simulink model "Simulatore_COMAU_UBHAND_IV_manipolazione_interna"  (Simulink 7.6)
settings: Solver=ode45  StartTime=0.0  StopTime=inf  RelTol=1e-3
values from neighbouring files (symbols this model uses):
  a_f = zeros(4,5)    [KinematicData.m]
  alpha_f = zeros(4,5)    [KinematicData.m]
  d_f = zeros(4,5)    [KinematicData.m]
  dc4_1 = 660    [KinematicData.m]
  T6HAND = [1 0 0 0;0 1 0 0; 0 0 1 357.48+95; 0 0 0 1]    [KinematicData.m]
  teta_d = atan(647.07/130)    [KinematicData.m]
  teta_f = zeros(4,5)    [KinematicData.m]
undefined symbols (not defined in the file or in its folder): a_c, a_w, alpha_c, alpha_w, d_c, d_w, Dt_fingers, teta_c
libraries: simulink x281, dspmtrx3 x171, aerolibutil x163, aerolibconvert2 x64, simulink_extras x46, vrlib x26, aerolibtransform2 x7, dspnetwork x1
(part 2 of 15)

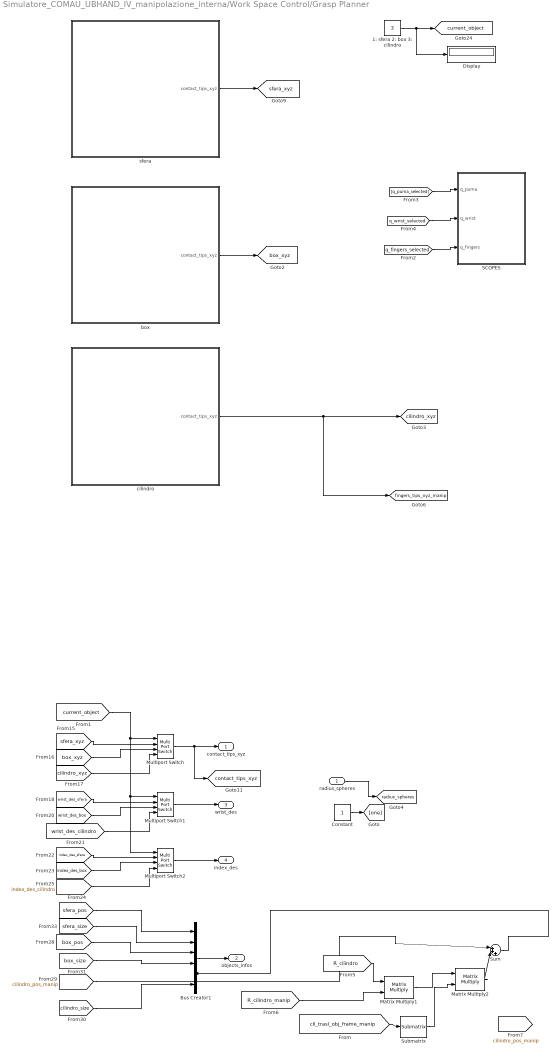
== system Simulatore_COMAU_UBHAND_IV_manipolazione_interna/Work Space Control/Grasp Planner ==
  radius_spheres : Inport
  1: sfera 2: box 3: cilindro : Constant  Value=3
  Bus Creator1 : BusCreator  Inputs=6
  Constant : Constant
  Display : Display
  Matrix Multiply1 : Product  Multiplication=Matrix(*)
  Matrix Multiply2 : Product  Multiplication=Matrix(*)
  Multiport Switch : MultiPortSwitch  zeroidx=off
  Multiport Switch1 : MultiPortSwitch  zeroidx=off
  Multiport Switch2 : MultiPortSwitch  zeroidx=off
  SCOPES : Subsystem
  Submatrix : dspmtrx3/Submatrix  RowSpan=Range of rows  RowStartMode=Index  RowStartIndex=1  RowEndMode=Index  RowEndIndex=3  ColSpan=One column  ColStartMode=Index  ColStartIndex=1  ColEndMode=Index  ColEndIndex=3
  Sum : Sum  Inputs=|+++
  box : Subsystem
  cilindro : Subsystem
  sfera : Subsystem
  contact_tips_xyz : Outport
  objects_infos : Outport  Port=2
  wrist_des : Outport  Port=3
  index_des : Outport  Port=4
  tag scope: box_xyz=global, cilindro_xyz=global, contact_tips_xyz=global, current_object=global, fingers_tips_xyz_manip=global, radius_spheres=global, sfera_xyz=global
  wires:
  Constant/1 -> [one]
  Multiport Switch/1 -> [contact_tips_xyz], contact_tips_xyz/1
  [sfera_xyz] -> Multiport Switch/2
  [box_xyz] -> Multiport Switch/3
  [cilindro_xyz] -> Multiport Switch/4
  radius_spheres/1 -> [radius_spheres]
  [sfera_pos] -> Bus Creator1/1
  [sfera_size] -> Bus Creator1/2
  [box_pos] -> Bus Creator1/3
  [box_size] -> Bus Creator1/4
  [cilindro_size] -> Bus Creator1/6
  Bus Creator1/1 -> objects_infos/1
  Multiport Switch1/1 -> wrist_des/1
  [wrist_des_sfera] -> Multiport Switch1/2
  [wrist_des_box] -> Multiport Switch1/3
  [wrist_des_cilindro] -> Multiport Switch1/4
  Multiport Switch2/1 -> index_des/1
  [index_des_sfera] -> Multiport Switch2/2
  [index_des_box] -> Multiport Switch2/3
  [index_des_cilindro] -> Multiport Switch2/4
  sfera/1(contact_tips_xyz) -> [sfera_xyz]
  box/1(contact_tips_xyz) -> [box_xyz]
  1: sfera 2: box 3: cilindro/1 -> [current_object], Display/1
  [current_object] -> Multiport Switch/1, Multiport Switch1/1, Multiport Switch2/1
  [q_puma_selected] -> SCOPES/1(q_puma)
  [q_wrist_selected] -> SCOPES/2(q_wrist)
  [q_fingers_selected] -> SCOPES/3(q_fingers)
  cilindro/1(contact_tips_xyz) -> [cilindro_xyz], [fingers_tips_xyz_manip]
  [cil_trasl_obj_frame_manip] -> Submatrix/1
  Matrix Multiply2/1 -> Sum/2
  [cilindro_pos_manip] -> Sum/1
  Sum/1 -> Bus Creator1/5
  Submatrix/1 -> Matrix Multiply2/2
  [R_cilindro_manip] -> Matrix Multiply1/2
  [R_cilindro] -> Matrix Multiply1/1
  Matrix Multiply1/1 -> Matrix Multiply2/1

== system Simulatore_COMAU_UBHAND_IV_manipolazione_interna/Work Space Control/Inverse_kinematic ==
  note: punti di contatto nel sistema di riferimento dito
  "fingers_tips_xyz " : Inport
  wrist_angles_des : Inport  Port=2
  index_angles_des : Inport  Port=3
  Demux : Demux  Outputs=5
  Demux1 : Demux  Outputs=20
  Display : Display
  Inverse_kinematic_comau : Subsystem
  Inverse_kinematic_hand : Subsystem
  Multiport Switch3 : MultiPortSwitch  Inputs=2  zeroidx=off
  Mux{,1,3..5} (5 alike) : Mux
  Mux2 : Mux  Inputs=5
  Mux6 : Mux  Inputs=5
  Submatrix : dspmtrx3/Submatrix  RowSpan=Range of rows  RowStartMode=Index  RowStartIndex=1  RowEndMode=Index  RowEndIndex=3  ColSpan=One column  ColStartMode=Index  ColStartIndex=1  ColEndMode=Index  ColEndIndex=3
  Tf0 : Subsystem
  teta[1..4] : Display
  teta[1..4]1 : Display
  wrist_angles_des1 : InportShadow  Port=2
  q[1..6] : Outport
  finger_joints references : Outport  Port=2
  T_0H : Outport  Port=3
  wires:
  Inverse_kinematic_comau/1(q[1..6]) -> q[1..6]/1
  wrist_angles_des/1 -> Inverse_kinematic_comau/2(wrist_angles_des)
  index_angles_des/1 -> Inverse_kinematic_comau/3(index_angles_des)
  Mux2/1 -> finger_joints references/1
  Inverse_kinematic_comau/2(T_0H) -> Tf0/1(T_0HAND), T_0H/1
  Tf0/1(fingers_references xyz fingers_references 1) -> [fingers_xyz_fing_ref], teta[1..4]1/1
  [fingers_xyz_fing_ref] -> Multiport Switch3/3
  Multiport Switch3/1 -> Demux/1
  Demux/1 -> Inverse_kinematic_hand/1(xyz_thumb)
  Inverse_kinematic_hand/1(Thumb) -> Mux2/1
  [fingers_tips_xyz] -> Multiport Switch3/2
  [fingers_tips_xyz] -> Tf0/3(fingers_references_xyz 0)
  "fingers_tips_xyz "/1 -> Demux1/1
  Demux1/1 -> Mux/1
  Demux1/2 -> Mux/2
  Demux1/3 -> Mux/3
  Demux1/4 -> Mux/4
  Demux1/5 -> Mux1/1
  Demux1/6 -> Mux1/2
  Demux1/7 -> Mux1/3
  Demux1/9 -> Mux3/1
  Demux1/10 -> Mux3/2
  Demux1/11 -> Mux3/3
  Demux1/12 -> Mux3/4
  Demux1/13 -> Mux4/1
  Demux1/14 -> Mux4/2
  Demux1/15 -> Mux4/3
  Demux1/16 -> Mux4/4
  Demux1/17 -> Mux5/1
  Demux1/18 -> Mux5/2
  Demux1/19 -> Mux5/3
  Demux1/20 -> Mux5/4
  Mux1/1 -> Mux6/2
  Mux/1 -> Mux6/1
  Mux6/1 -> [fingers_tips_xyz]
  wrist_angles_des1/1 -> Tf0/2(wrist_angles[1..2])
  [reference_mode] -> Multiport Switch3/1
  Mux3/1 -> Mux6/3
  Mux4/1 -> Mux6/4
  Mux5/1 -> Mux6/5
  Demux/5 -> Inverse_kinematic_hand/5(xyz_little)
  Demux/4 -> Inverse_kinematic_hand/4(xyz_ring)
  Demux/3 -> Inverse_kinematic_hand/3(xyz_middle)
  Demux/2 -> Inverse_kinematic_hand/2(xyz_index)
  Demux1/8 -> Mux1/4
  Submatrix/1 -> Inverse_kinematic_comau/1(end_effector_xyz), Display/1
  Inverse_kinematic_hand/5(Little) -> Mux2/5
  Inverse_kinematic_hand/4(Ring) -> Mux2/4
  Inverse_kinematic_hand/3(Middle) -> Mux2/3
  Inverse_kinematic_hand/2(Index) -> Mux2/2, teta[1..4]/1
  [index_xyz_0] -> Submatrix/1

== system Simulatore_COMAU_UBHAND_IV_manipolazione_interna/Work Space Control/Piano di lavoro ==
  Constant : Constant
  Mux1 : Mux  Inputs=3
  Mux2 : Mux  Inputs=3
  x : simulink/Math Operations/Slider Gain  low=-1000  gain=1600  high=2000
  x_size : simulink/Math Operations/Slider Gain  low=-1000  gain=3000  high=3000
  y : simulink/Math Operations/Slider Gain  low=-1000  gain=-120  high=1000
  y_size : simulink/Math Operations/Slider Gain  low=-1000  gain=40  high=1000
  z : simulink/Math Operations/Slider Gain  low=-1000  gain=250  high=5010
  z_size : simulink/Math Operations/Slider Gain  low=-1000  gain=1800  high=5010
  tag scope: piano_size=global, piano_xyz=global
  wires:
  [one] -> x/1
  x/1 -> Mux2/1
  [one] -> y/1
  [one] -> z/1
  z/1 -> Mux2/3
  y/1 -> Mux2/2
  [one] -> x_size/1
  x_size/1 -> Mux1/1
  [one] -> y_size/1
  [one] -> z_size/1
  z_size/1 -> Mux1/3
  y_size/1 -> Mux1/2
  Mux2/1 -> [piano_xyz]
  Mux1/1 -> [piano_size]
  Constant/1 -> [one]

== system Simulatore_COMAU_UBHAND_IV_manipolazione_interna/Work Space Control/R_end_effector ==
  alpha,beta,gamma : Inport
  Demux : Demux  Outputs=3
  Matrix Multiply3 : Product  Multiplication=Matrix(*)
  Matrix Multiply4 : Product  Multiplication=Matrix(*)
  rot_x : Subsystem
  rot_y : Subsystem
  rot_z : Subsystem
  R_end_effector : Outport
  wires:
  Matrix Multiply4/1 -> R_end_effector/1
  Matrix Multiply3/1 -> Matrix Multiply4/1
  rot_z/1(rotz) -> Matrix Multiply4/2
  rot_y/1(roty) -> Matrix Multiply3/2
  rot_x/1(rotx) -> Matrix Multiply3/1
  Demux/1 -> rot_x/1(alpha)
  Demux/2 -> rot_y/1(beta)
  Demux/3 -> rot_z/1(gamma)
  alpha,beta,gamma/1 -> Demux/1

== system Simulatore_COMAU_UBHAND_IV_manipolazione_interna/Work Space Control/Step Signals fingers_wrist ==
  All_fingers_together_back_forward : Constant  Value=80
  All_fingers_together_medial : Constant  Value=80
  All_fingers_together_proximal_left_right : Constant  Value=0
  Index_medial1 : Constant  Value=30
  Index_proximal_back_forward1 : Constant  Value=45
  Index_proximal_left_right1 : Constant  Value=0
  Little_medial : Constant  Value=0
  Little_proximal_back_forward : Constant  Value=0
  Little_proximal_left_right : Constant  Value=0
  Middle_medial : Constant  Value=30
  Middle_proximal_back_forward : Constant  Value=45
  Middle_proximal_left_right : Constant  Value=0
  Mux{1..5,7} (6 alike) : Mux  Inputs=3
  Mux8 : Mux  Inputs=5
  Mux9 : Mux  Inputs=5
  Ring_medial : Constant  Value=0
  Ring_proximal_back_forward : Constant  Value=0
  Ring_proximal_left_right : Constant  Value=0
  Switch wrist : simulink/Signal Routing/Manual Switch  sw=0  action=0  varsize=off
  Thumb_medial : Constant  Value=50
  Thumb_proximal_back_forward : Constant  Value=50
  Thumb_proximal_left_right : Constant  Value=50
  fingers_angles : Outport
  wires:
  Middle_proximal_left_right/1 -> Mux3/1
  Middle_medial/1 -> Mux3/3
  Middle_proximal_back_forward/1 -> Mux3/2
  Ring_proximal_left_right/1 -> Mux4/1
  Ring_medial/1 -> Mux4/3
  Ring_proximal_back_forward/1 -> Mux4/2
  Little_proximal_left_right/1 -> Mux5/1
  Little_medial/1 -> Mux5/3
  Little_proximal_back_forward/1 -> Mux5/2
  Mux1/1 -> [thumb], Mux9/1
  Thumb_proximal_left_right/1 -> Mux1/1
  Thumb_medial/1 -> Mux1/3
  Thumb_proximal_back_forward/1 -> Mux1/2
  Mux3/1 -> [index]
  Mux4/1 -> [ring]
  Mux5/1 -> [little]
  Mux7/1 -> Mux9/3, Mux9/2, Mux9/4, Mux9/5
  All_fingers_together_proximal_left_right/1 -> Mux7/1
  All_fingers_together_medial/1 -> Mux7/3
  All_fingers_together_back_forward/1 -> Mux7/2
  Mux9/1 -> Switch wrist/2
  Mux8/1 -> Switch wrist/1
  Switch wrist/1 -> fingers_angles/1
  [thumb] -> Mux8/1
  Index_proximal_left_right1/1 -> Mux2/1
  Index_medial1/1 -> Mux2/3
  Index_proximal_back_forward1/1 -> Mux2/2
  Mux2/1 -> [middle]
  [little] -> Mux8/5
  [ring] -> Mux8/4
  [middle] -> Mux8/3
  [index] -> Mux8/2

== system Simulatore_COMAU_UBHAND_IV_manipolazione_interna/Work Space Control/posa_selection ==
  Index_medial_riposo : Constant  Value=0
  Index_proximal_back_forward_riposo : Constant  Value=0
  Index_proximal_left_right_riposo : Constant  Value=0
  Multiport Switch{,1..3} (4 alike) : MultiPortSwitch  Inputs=2  zeroidx=off
  Mux : Mux  Inputs=5
  Mux3 : Mux  Inputs=2
  Mux4 : Mux  Inputs=3
  Mux5 : Mux  Inputs=5
  fingers_rest_angles : Constant  Value=[ 0 0 0]
  wrist_back_forward_riposo : simulink/Math Operations/Slider Gain  low=-180  gain=0  high=180
  wrist_left_right_riposo : simulink/Math Operations/Slider Gain  low=-180  gain=0  high=180
  q_wrist : Outport
  q_fingers : Outport  Port=2
  tag scope: index_angles_des_C=global, wrist_angles_des_C=global
  wires:
  [one] -> wrist_left_right_riposo/1
  wrist_left_right_riposo/1 -> Mux3/1
  [one] -> wrist_back_forward_riposo/1
  wrist_back_forward_riposo/1 -> Mux3/2
  Index_proximal_left_right_riposo/1 -> Mux4/1
  Index_medial_riposo/1 -> Mux4/3
  Index_proximal_back_forward_riposo/1 -> Mux4/2
  [q_thumb] -> Mux/1
  [q_middle] -> Mux/3
  [q_ring] -> Mux/4
  [q_little] -> Mux/5
  fingers_rest_angles/1 -> Mux5/1, Mux5/3, Mux5/4, Mux5/5
  Mux4/1 -> Mux5/2
  Mux/1 -> Multiport Switch1/2
  Mux5/1 -> Multiport Switch1/3
  Multiport Switch1/1 -> q_fingers/1
  Mux3/1 -> Multiport Switch/3
  Multiport Switch/1 -> q_wrist/1
  [wrist_angles_des_C_2] -> Multiport Switch2/3
  [wrist_angles_des_C_1] -> Multiport Switch2/2
  Multiport Switch2/1 -> Multiport Switch/2, [wrist_angles_des_C]
  [index_angles_des_C_1] -> Multiport Switch3/2
  Multiport Switch3/1 -> [index_angles_des_C]
  [reference_mode] -> Multiport Switch2/1
  [reference_mode] -> Multiport Switch3/1
  [index_angles_des_C_2] -> Multiport Switch3/3
  [posa_selection] -> Multiport Switch/1, Multiport Switch1/1
  [q_index] -> Mux/2

== system Simulatore_COMAU_UBHAND_IV_manipolazione_interna/Work Space Control/wrist index angles des2 ==
  Constant : Constant
  Index_medial_des : simulink/Math Operations/Slider Gain  low=0  gain=30  high=110
  Index_proximal_back_forward_des : simulink/Math Operations/Slider Gain  low=0  gain=15  high=90
  Index_proximal_left_right_des : simulink/Math Operations/Slider Gain  low=-180  gain=20  high=180
  Mux1 : Mux  Inputs=2
  Mux2 : Mux  Inputs=3
  wrist_back_forward_des : simulink/Math Operations/Slider Gain  low=-180  gain=0  high=180
  wrist_left_right_des : simulink/Math Operations/Slider Gain  low=-180  gain=0  high=180
  wrist_des : Outport
  index_des : Outport  Port=2
  wires:
  Constant/1 -> [one]
  Mux1/1 -> wrist_des/1
  Mux2/1 -> index_des/1
  Index_proximal_back_forward_des/1 -> Mux2/2
  Index_medial_des/1 -> Mux2/3
  Index_proximal_left_right_des/1 -> Mux2/1
  wrist_back_forward_des/1 -> Mux1/2
  [one] -> wrist_back_forward_des/1
  wrist_left_right_des/1 -> Mux1/1
  [one] -> wrist_left_right_des/1
  [one] -> Index_proximal_left_right_des/1
  [one] -> Index_proximal_back_forward_des/1
  [one] -> Index_medial_des/1

== system Simulatore_COMAU_UBHAND_IV_manipolazione_interna/Joint Space Control/Direct_kinematic2/Direct_kinematic_Comau ==
  q[1..6] : Inport
  Demux3 : Demux  Outputs=6
  Matrix Multiply : Product  Multiplication=Matrix(*)
  Mux : Mux  Inputs=3
  Mux2 : Mux  Inputs=3
  T_0_3 : Subsystem
  T_3_6 : Subsystem
  T_0_6 : Outport
  wires:
  Demux3/1 -> Mux/1
  Demux3/2 -> Mux/2
  Demux3/3 -> Mux/3
  Mux/1 -> T_0_3/1(q[1..3])
  Demux3/4 -> Mux2/1
  Demux3/5 -> Mux2/2
  Demux3/6 -> Mux2/3
  Mux2/1 -> T_3_6/1(q[1..3])
  T_3_6/1(T_3_6) -> Matrix Multiply/2
  q[1..6]/1 -> Demux3/1
  T_0_3/1(T_0_3) -> Matrix Multiply/1
  Matrix Multiply/1 -> T_0_6/1

== system Simulatore_COMAU_UBHAND_IV_manipolazione_interna/Joint Space Control/Direct_kinematic2/Direct_kinematic_hand ==
  Joint_references : Inport
  T_08 : Inport  Port=2
  Constant : Constant  Value=Dt_fingers(1,1).T_w2_finger_base  VectorParams1D=off
  Constant1 : Constant  Value=Dt_fingers(1,2).T_w2_finger_base  VectorParams1D=off
  Constant2 : Constant  Value=Dt_fingers(1,3).T_w2_finger_base  VectorParams1D=off
  Constant3 : Constant  Value=Dt_fingers(1,4).T_w2_finger_base  VectorParams1D=off
  Constant4 : Constant  Value=Dt_fingers(1,5).T_w2_finger_base  VectorParams1D=off
  Demux : Demux  Outputs=5
  Direct_kinematic_index : Subsystem
  Direct_kinematic_little : Subsystem
  Direct_kinematic_middle : Subsystem
  Direct_kinematic_ring : Subsystem
  Direct_kinematic_thumb : Subsystem
  Matrix Multiply{,1..4} (5 alike) : Product  Multiplication=Matrix(*)
  xyz{,1..4} (5 alike) : dspmtrx3/Submatrix  RowSpan=Range of rows  RowStartMode=Index  RowStartIndex=1  RowEndMode=Index  RowEndIndex=4  ColSpan=One column  ColStartMode=Index  ColStartIndex=4  ColEndMode=Index  ColEndIndex=3
  T_finger_tip_thumb : Outport
  T_finger_tip_index : Outport  Port=2
  T_finger_tip_middle : Outport  Port=3
  T_finger_tip_ring : Outport  Port=4
  T_finger_tip_little : Outport  Port=5
  wires:
  T_08/1 -> Matrix Multiply/1, Matrix Multiply1/1, Matrix Multiply2/1, Matrix Multiply3/1, Matrix Multiply4/1
  Constant/1 -> Matrix Multiply/2
  Constant1/1 -> Matrix Multiply1/2
  Constant2/1 -> Matrix Multiply2/2
  Constant3/1 -> Matrix Multiply3/2
  Constant4/1 -> Matrix Multiply4/2
  Matrix Multiply/1 -> Direct_kinematic_thumb/2(T_0f)
  Matrix Multiply1/1 -> Direct_kinematic_index/2(T_0f)
  Matrix Multiply2/1 -> Direct_kinematic_middle/2(T_0f)
  Matrix Multiply3/1 -> Direct_kinematic_ring/2(T_0f)
  Matrix Multiply4/1 -> Direct_kinematic_little/2(T_0f)
  Joint_references/1 -> Demux/1
  Demux/1 -> Direct_kinematic_thumb/1(Joints_reference)
  Demux/2 -> Direct_kinematic_index/1(Joints_reference)
  Demux/3 -> Direct_kinematic_middle/1(Joints_reference)
  Demux/4 -> Direct_kinematic_ring/1(Joints_reference)
  Demux/5 -> Direct_kinematic_little/1(Joints_reference)
  Direct_kinematic_index/1(T_finger_tip) -> xyz1/1
  Direct_kinematic_middle/1(T_finger_tip) -> xyz2/1
  Direct_kinematic_ring/1(T_finger_tip) -> xyz3/1
  Direct_kinematic_little/1(T_finger_tip) -> xyz4/1
  Direct_kinematic_thumb/1(T_finger_tip) -> xyz/1
  xyz1/1 -> T_finger_tip_index/1
  xyz/1 -> T_finger_tip_thumb/1
  xyz2/1 -> T_finger_tip_middle/1
  xyz3/1 -> T_finger_tip_ring/1
  xyz4/1 -> T_finger_tip_little/1

== system Simulatore_COMAU_UBHAND_IV_manipolazione_interna/Joint Space Control/Direct_kinematic2/Direct_kinematic_wrist ==
  wrist_angles[1..2] : Inport
  T_0HAND : Inport  Port=2
  Demux3 : Demux  Outputs=2
  Mux : Mux  Inputs=2
  T_0_8 : Subsystem
  T_08 : Outport
  wires:
  T_0_8/1(T_08) -> T_08/1
  wrist_angles[1..2]/1 -> Demux3/1
  Mux/1 -> T_0_8/1(wrist_angles[1..2])
  Demux3/2 -> Mux/2
  Demux3/1 -> Mux/1
  T_0HAND/1 -> T_0_8/2(T_0HAND)

== system Simulatore_COMAU_UBHAND_IV_manipolazione_interna/SCOPES/Direct_kinematic2/Direct_kinematic_Comau ==
  q[1..6] : Inport
  Demux3 : Demux  Outputs=6
  Matrix Multiply : Product  Multiplication=Matrix(*)
  Mux : Mux  Inputs=3
  Mux2 : Mux  Inputs=3
  T_0_3 : Subsystem
  T_3_6 : Subsystem
  T_0_6 : Outport
  wires:
  Demux3/1 -> Mux/1
  Demux3/2 -> Mux/2
  Demux3/3 -> Mux/3
  Mux/1 -> T_0_3/1(q[1..3])
  Demux3/4 -> Mux2/1
  Demux3/5 -> Mux2/2
  Demux3/6 -> Mux2/3
  Mux2/1 -> T_3_6/1(q[1..3])
  T_3_6/1(T_3_6) -> Matrix Multiply/2
  q[1..6]/1 -> Demux3/1
  T_0_3/1(T_0_3) -> Matrix Multiply/1
  Matrix Multiply/1 -> T_0_6/1

== system Simulatore_COMAU_UBHAND_IV_manipolazione_interna/SCOPES/Direct_kinematic2/Direct_kinematic_hand ==
  Joint_references : Inport
  T_08 : Inport  Port=2
  Constant : Constant  Value=Dt_fingers(1,1).T_w2_finger_base  VectorParams1D=off
  Constant1 : Constant  Value=Dt_fingers(1,2).T_w2_finger_base  VectorParams1D=off
  Constant2 : Constant  Value=Dt_fingers(1,3).T_w2_finger_base  VectorParams1D=off
  Constant3 : Constant  Value=Dt_fingers(1,4).T_w2_finger_base  VectorParams1D=off
  Constant4 : Constant  Value=Dt_fingers(1,5).T_w2_finger_base  VectorParams1D=off
  Demux : Demux  Outputs=5
  Direct_kinematic_index : Subsystem
  Direct_kinematic_little : Subsystem
  Direct_kinematic_middle : Subsystem
  Direct_kinematic_ring : Subsystem
  Direct_kinematic_thumb : Subsystem
  Matrix Multiply{,1..4} (5 alike) : Product  Multiplication=Matrix(*)
  xyz{,1..4} (5 alike) : dspmtrx3/Submatrix  RowSpan=Range of rows  RowStartMode=Index  RowStartIndex=1  RowEndMode=Index  RowEndIndex=4  ColSpan=One column  ColStartMode=Index  ColStartIndex=4  ColEndMode=Index  ColEndIndex=3
  T_finger_tip_thumb : Outport
  T_finger_tip_index : Outport  Port=2
  T_finger_tip_middle : Outport  Port=3
  T_finger_tip_ring : Outport  Port=4
  T_finger_tip_little : Outport  Port=5
  T_thumb_base : Outport  Port=6
  tag scope: T_0_little=global, T_0_middle=global, T_0_ring=global, T_0_thumb=global
  wires:
  T_08/1 -> Matrix Multiply/1, Matrix Multiply1/1, Matrix Multiply2/1, Matrix Multiply3/1, Matrix Multiply4/1
  Constant/1 -> Matrix Multiply/2
  Constant1/1 -> Matrix Multiply1/2
  Constant2/1 -> Matrix Multiply2/2
  Constant3/1 -> Matrix Multiply3/2
  Constant4/1 -> Matrix Multiply4/2
  Matrix Multiply/1 -> Direct_kinematic_thumb/2(T_0f), [T_0_thumb]
  Matrix Multiply1/1 -> Direct_kinematic_index/2(T_0f)
  Matrix Multiply2/1 -> Direct_kinematic_middle/2(T_0f), [T_0_middle]
  Matrix Multiply3/1 -> Direct_kinematic_ring/2(T_0f), [T_0_ring]
  Matrix Multiply4/1 -> Direct_kinematic_little/2(T_0f), [T_0_little]
  Joint_references/1 -> Demux/1
  Demux/1 -> Direct_kinematic_thumb/1(Joints_reference)
  Demux/2 -> Direct_kinematic_index/1(Joints_reference)
  Demux/3 -> Direct_kinematic_middle/1(Joints_reference)
  Demux/4 -> Direct_kinematic_ring/1(Joints_reference)
  Demux/5 -> Direct_kinematic_little/1(Joints_reference)
  Direct_kinematic_index/1(T_finger_tip) -> xyz1/1
  Direct_kinematic_middle/1(T_finger_tip) -> xyz2/1
  Direct_kinematic_ring/1(T_finger_tip) -> xyz3/1
  Direct_kinematic_little/1(T_finger_tip) -> xyz4/1
  Direct_kinematic_thumb/1(T_finger_tip) -> xyz/1
  Direct_kinematic_thumb/2(T_thumb_base) -> T_thumb_base/1
  xyz1/1 -> T_finger_tip_index/1
  xyz/1 -> T_finger_tip_thumb/1
  xyz2/1 -> T_finger_tip_middle/1
  xyz3/1 -> T_finger_tip_ring/1
  xyz4/1 -> T_finger_tip_little/1

== system Simulatore_COMAU_UBHAND_IV_manipolazione_interna/SCOPES/Direct_kinematic2/Direct_kinematic_wrist ==
  wrist_angles[1..2] : Inport
  T_0HAND : Inport  Port=2
  Demux3 : Demux  Outputs=2
  Mux : Mux  Inputs=2
  T_0_8 : Subsystem
  T_08 : Outport
  wires:
  T_0_8/1(T_08) -> T_08/1
  wrist_angles[1..2]/1 -> Demux3/1
  Mux/1 -> T_0_8/1(wrist_angles[1..2])
  Demux3/2 -> Mux/2
  Demux3/1 -> Mux/1
  T_0HAND/1 -> T_0_8/2(T_0HAND)

== system Simulatore_COMAU_UBHAND_IV_manipolazione_interna/VISUALIZATION/Subsystem/sphere_xyz1 ==
  xyz_0 : Inport
  Constant : Constant  Value=0
  Constant1 : Constant  Value=0
  Constant2 : Constant  Value=0
  Demux : Demux  Outputs=3
  Display : Display
  Display1 : Display
  Gain : Gain  Gain=-1
  Gain1 : Gain  Gain=-1
  Gain2 : Gain
  Mux : Mux  Inputs=3
  Sum : Sum
  Sum1 : Sum
  Sum2 : Sum
  xyz_vrl : Outport
  wires:
  xyz_0/1 -> Display1/1, Demux/1
  Demux/1 -> Sum/2
  Constant/1 -> Sum/1
  Demux/3 -> Sum2/2
  Constant2/1 -> Sum2/1
  Demux/2 -> Sum1/2
  Constant1/1 -> Sum1/1
  Mux/1 -> xyz_vrl/1, Display/1
  Sum/1 -> Gain/1
  Sum1/1 -> Gain1/1
  Sum2/1 -> Gain2/1
  Gain/1 -> Mux/3
  Gain2/1 -> Mux/2
  Gain1/1 -> Mux/1

== system Simulatore_COMAU_UBHAND_IV_manipolazione_interna/VISUALIZATION/Subsystem1/box_xyz ==
  xyz_0 : Inport
  Constant : Constant  Value=0
  Constant1 : Constant  Value=0
  Constant2 : Constant  Value=0
  Demux : Demux  Outputs=3
  Display : Display
  Display1 : Display
  Gain : Gain  Gain=-1
  Gain1 : Gain  Gain=-1
  Gain2 : Gain
  Mux : Mux  Inputs=3
  Sum : Sum
  Sum1 : Sum
  Sum2 : Sum
  xyz_vrl : Outport
  wires:
  xyz_0/1 -> Display1/1, Demux/1
  Demux/1 -> Sum/2
  Constant/1 -> Sum/1
  Demux/3 -> Sum2/2
  Constant2/1 -> Sum2/1
  Demux/2 -> Sum1/2
  Constant1/1 -> Sum1/1
  Mux/1 -> xyz_vrl/1, Display/1
  Sum/1 -> Gain/1
  Sum1/1 -> Gain1/1
  Sum2/1 -> Gain2/1
  Gain/1 -> Mux/3
  Gain2/1 -> Mux/2
  Gain1/1 -> Mux/1

== system Simulatore_COMAU_UBHAND_IV_manipolazione_interna/VISUALIZATION/Subsystem1/cilindro_xyz ==
  xyz_0 : Inport
  Constant : Constant  Value=0
  Constant1 : Constant  Value=0
  Constant2 : Constant  Value=0
  Demux : Demux  Outputs=3
  Display : Display
  Display1 : Display
  Gain : Gain  Gain=-1
  Gain1 : Gain  Gain=-1
  Gain2 : Gain
  Mux : Mux  Inputs=3
  Sum : Sum
  Sum1 : Sum
  Sum2 : Sum
  xyz_vrl : Outport
  wires:
  Gain1/1 -> Mux/1
  Gain2/1 -> Mux/2
  Gain/1 -> Mux/3
  Sum2/1 -> Gain2/1
  Sum1/1 -> Gain1/1
  Sum/1 -> Gain/1
  Mux/1 -> Display/1, xyz_vrl/1
  Constant1/1 -> Sum1/1
  Demux/2 -> Sum1/2
  Constant2/1 -> Sum2/1
  Demux/3 -> Sum2/2
  Constant/1 -> Sum/1
  Demux/1 -> Sum/2
  xyz_0/1 -> Demux/1, Display1/1

== system Simulatore_COMAU_UBHAND_IV_manipolazione_interna/VISUALIZATION/Subsystem1/sphere_xyz ==
  xyz_0 : Inport
  Constant : Constant  Value=0
  Constant1 : Constant  Value=0
  Constant2 : Constant  Value=0
  Demux : Demux  Outputs=3
  Display : Display
  Display1 : Display
  Gain : Gain  Gain=-1
  Gain1 : Gain  Gain=-1
  Gain2 : Gain
  Mux : Mux  Inputs=3
  Sum : Sum
  Sum1 : Sum
  Sum2 : Sum
  xyz_vrl : Outport
  wires:
  Gain1/1 -> Mux/1
  Gain2/1 -> Mux/2
  Gain/1 -> Mux/3
  Sum2/1 -> Gain2/1
  Sum1/1 -> Gain1/1
  Sum/1 -> Gain/1
  Mux/1 -> Display/1, xyz_vrl/1
  Constant1/1 -> Sum1/1
  Demux/2 -> Sum1/2
  Constant2/1 -> Sum2/1
  Demux/3 -> Sum2/2
  Constant/1 -> Sum/1
  Demux/1 -> Sum/2
  xyz_0/1 -> Demux/1, Display1/1

== system Simulatore_COMAU_UBHAND_IV_manipolazione_interna/VISUALIZATION/Subsystem3/Subsystem1 ==
  TOHAND : Inport
  Demux8 : Demux
  Demux9 : Demux
  Gain : Gain  Gain=-1
  Gain1 : Gain  Gain=-1
  Mux : Mux
  Mux1 : Mux
  Rotation Matrix to VRML Rotation : vrlib/Utilities/Rotation Matrix to VRML Rotation  maxzero=1e-12
  Submatrix : dspmtrx3/Submatrix  RowSpan=Range of rows  RowStartMode=Index  RowStartIndex=1  RowEndMode=Index  RowEndIndex=3  ColSpan=One column  ColStartMode=Index  ColStartIndex=4  ColEndMode=Last  ColEndIndex=1
  Submatrix1 : dspmtrx3/Submatrix  RowSpan=Range of rows  RowStartMode=Index  RowStartIndex=1  RowEndMode=Index  RowEndIndex=3  ColSpan=Range of columns  ColStartMode=Index  ColStartIndex=1  ColEndMode=Index  ColEndIndex=3
  Wrist : Subsystem
  ax/angle_vrl_wrist : Outport
  xyz_vrl_wrist : Outport  Port=2
  wires:
  Submatrix1/1 -> Rotation Matrix to VRML Rotation/1
  Submatrix/1 -> Wrist/1(xyz_0)
  TOHAND/1 -> Submatrix1/1, Submatrix/1
  Gain1/1 -> Mux/1
  Gain/1 -> Mux/3
  Demux8/2 -> Gain1/1
  Demux8/1 -> Gain/1
  Mux1/1 -> ax/angle_vrl_wrist/1
  Demux9/4 -> Mux1/4
  Demux9/3 -> Mux1/3
  Demux9/2 -> Mux1/2
  Demux9/1 -> Mux1/1
  Mux/1 -> Demux9/1
  Demux8/3 -> Mux/2
  Demux8/4 -> Mux/4
  Rotation Matrix to VRML Rotation/1 -> Demux8/1
  Wrist/1(xyz_vrl) -> xyz_vrl_wrist/1

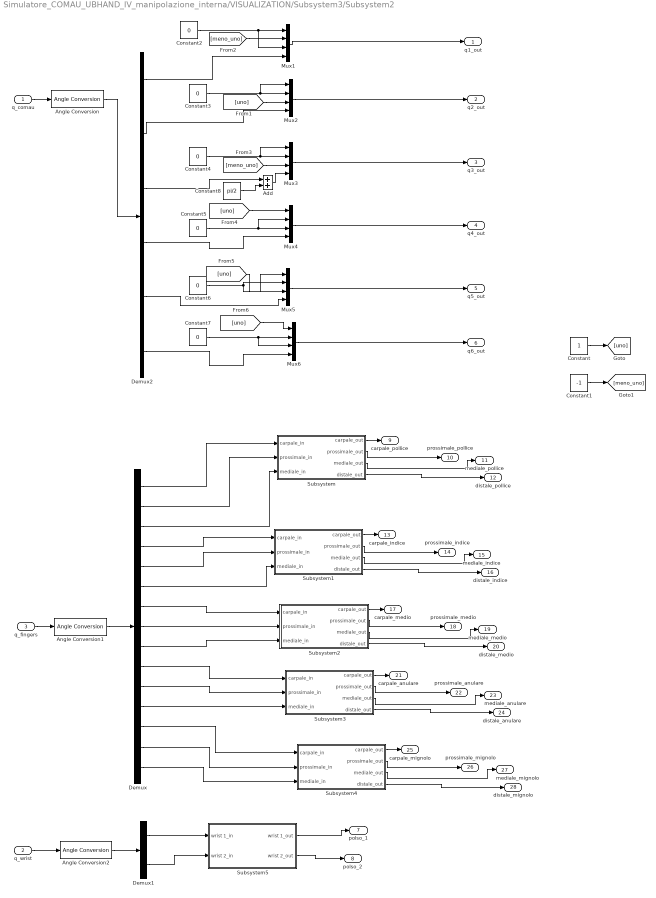
== system Simulatore_COMAU_UBHAND_IV_manipolazione_interna/VISUALIZATION/Subsystem3/Subsystem2 ==
  q_comau : Inport
  q_wrist : Inport  Port=2
  q_fingers : Inport  Port=3
  Add : Sum
  Angle Conversion : aerolibconvert2/Angle Conversion  IU=deg  OU=rad
  Angle Conversion1 : aerolibconvert2/Angle Conversion  IU=deg  OU=rad
  Angle Conversion2 : aerolibconvert2/Angle Conversion  IU=deg  OU=rad
  Constant : Constant
  Constant1 : Constant  Value=-1
  Constant{2..7} (6 alike) : Constant  Value=0
  Constant8 : Constant  Value=pi/2
  Demux : Demux  Outputs=15
  Demux1 : Demux  Outputs=2
  Demux2 : Demux  Outputs=6
  Mux{1..6} (6 alike) : Mux
  Subsystem : Subsystem
  Subsystem1 : Subsystem
  Subsystem2 : Subsystem
  Subsystem3 : Subsystem
  Subsystem4 : Subsystem
  Subsystem5 : Subsystem
  q1_out : Outport
  q2_out : Outport  Port=2
  q3_out : Outport  Port=3
  q4_out : Outport  Port=4
  q5_out : Outport  Port=5
  q6_out : Outport  Port=6
  polso_1 : Outport  Port=7
  polso_2 : Outport  Port=8
  carpale_pollice : Outport  Port=9
  prossimale_pollice : Outport  Port=10
  mediale_pollice : Outport  Port=11
  distale_pollice : Outport  Port=12
  carpale_indice : Outport  Port=13
  prossimale_indice : Outport  Port=14
  mediale_indice : Outport  Port=15
  distale_indice : Outport  Port=16
  carpale_medio : Outport  Port=17
  prossimale_medio : Outport  Port=18
  mediale_medio : Outport  Port=19
  distale_medio : Outport  Port=20
  carpale_anulare : Outport  Port=21
  prossimale_anulare : Outport  Port=22
  mediale_anulare : Outport  Port=23
  distale_anulare : Outport  Port=24
  carpale_mignolo : Outport  Port=25
  prossimale_mignolo : Outport  Port=26
  mediale_mignolo : Outport  Port=27
  distale_mignolo : Outport  Port=28
  wires:
  Subsystem/1(carpale_out) -> carpale_pollice/1
  Subsystem/2(prossimale_out) -> prossimale_pollice/1
  Subsystem/3(mediale_out) -> mediale_pollice/1
  Subsystem/4(distale_out) -> distale_pollice/1
  q_fingers/1 -> Angle Conversion1/1
  Demux/1 -> Subsystem/1(carpale_in)
  Demux/2 -> Subsystem/2(prossimale_in)
  Demux/3 -> Subsystem/3(mediale_in)
  Subsystem1/1(carpale_out) -> carpale_indice/1
  Subsystem1/2(prossimale_out) -> prossimale_indice/1
  Subsystem1/3(mediale_out) -> mediale_indice/1
  Subsystem1/4(distale_out) -> distale_indice/1
  Subsystem2/1(carpale_out) -> carpale_medio/1
  Subsystem2/2(prossimale_out) -> prossimale_medio/1
  Subsystem2/3(mediale_out) -> mediale_medio/1
  Subsystem2/4(distale_out) -> distale_medio/1
  Subsystem3/1(carpale_out) -> carpale_anulare/1
  Subsystem3/2(prossimale_out) -> prossimale_anulare/1
  Subsystem3/3(mediale_out) -> mediale_anulare/1
  Subsystem3/4(distale_out) -> distale_anulare/1
  Subsystem4/1(carpale_out) -> carpale_mignolo/1
  Subsystem4/2(prossimale_out) -> prossimale_mignolo/1
  Subsystem4/3(mediale_out) -> mediale_mignolo/1
  Subsystem4/4(distale_out) -> distale_mignolo/1
  Demux/4 -> Subsystem1/1(carpale_in)
  Demux/5 -> Subsystem1/2(prossimale_in)
  Demux/6 -> Subsystem1/3(mediale_in)
  q_wrist/1 -> Angle Conversion2/1
  Demux1/1 -> Subsystem5/1(wrist 1_in)
  Demux1/2 -> Subsystem5/2(wrist 2_in)
  Subsystem5/1(wrist 1_out) -> polso_1/1
  Subsystem5/2(wrist 2_out) -> polso_2/1
  Demux/7 -> Subsystem2/1(carpale_in)
  Demux/8 -> Subsystem2/2(prossimale_in)
  Demux/9 -> Subsystem2/3(mediale_in)
  Demux/10 -> Subsystem3/1(carpale_in)
  Demux/11 -> Subsystem3/2(prossimale_in)
  Demux/12 -> Subsystem3/3(mediale_in)
  Demux/13 -> Subsystem4/1(carpale_in)
  Demux/14 -> Subsystem4/2(prossimale_in)
  Demux/15 -> Subsystem4/3(mediale_in)
  Mux1/1 -> q1_out/1
  Constant2/1 -> Mux1/1, Mux1/3
  Mux2/1 -> q2_out/1
  Constant3/1 -> Mux2/1, Mux2/2
  Demux2/2 -> Mux2/4
  [uno] -> Mux2/3
  Mux3/1 -> q3_out/1
  Constant4/1 -> Mux3/2, Mux3/1
  [meno_uno] -> Mux3/3
  Mux4/1 -> q4_out/1
  Constant5/1 -> Mux4/3, Mux4/2
  Demux2/4 -> Mux4/4
  Mux5/1 -> q5_out/1
  Mux6/1 -> q6_out/1
  Constant7/1 -> Mux6/3, Mux6/2
  Demux2/6 -> Mux6/4
  [meno_uno] -> Mux1/2
  Constant6/1 -> Mux5/1, Mux5/2
  [uno] -> Mux4/1
  [uno] -> Mux5/3
  [uno] -> Mux6/1
  Demux2/3 -> Add/1
  Constant8/1 -> Add/2
  Add/1 -> Mux3/4
  q_comau/1 -> Angle Conversion/1
  Angle Conversion/1 -> Demux2/1
  Demux2/5 -> Mux5/4
  Demux2/1 -> Mux1/4
  Constant/1 -> [uno]
  Constant1/1 -> [meno_uno]
  Angle Conversion1/1 -> Demux/1
  Angle Conversion2/1 -> Demux1/1

== system Simulatore_COMAU_UBHAND_IV_manipolazione_interna/VISUALIZATION/Subsystem3/finger_tip_xyz ==
  T_finger_tip_thumb : Inport  PortDimensions=4
  T_finger_tip_index : Inport  Port=2  PortDimensions=4
  T_finger_tip_middle : Inport  Port=3  PortDimensions=4
  T_finger_tip_ring : Inport  Port=4  PortDimensions=4
  T_finger_tip_little : Inport  Port=5  PortDimensions=4
  Submatrix{,1..4} (5 alike) : dspmtrx3/Submatrix  RowSpan=Range of rows  RowStartMode=Index  RowStartIndex=1  RowEndMode=Index  RowEndIndex=3  ColSpan=One column  ColStartMode=First  ColStartIndex=4  ColEndMode=Index  ColEndIndex=3
  world_frame2vrl_frame : Subsystem
  world_frame2vrl_frame1 : Subsystem
  world_frame2vrl_frame2 : Subsystem
  world_frame2vrl_frame3 : Subsystem
  world_frame2vrl_frame4 : Subsystem
  vrl_xyz_thumb : Outport
  vrl_xyz_index : Outport  Port=2
  vrl_xyz_middle : Outport  Port=3
  vrl_xyz_ring : Outport  Port=4
  vrl_xyz_little : Outport  Port=5
  wires:
  Submatrix/1 -> world_frame2vrl_frame/1(xyz_0)
  T_finger_tip_thumb/1 -> Submatrix/1
  world_frame2vrl_frame/1(xyz_vrl) -> vrl_xyz_thumb/1
  Submatrix1/1 -> world_frame2vrl_frame1/1(xyz_0)
  T_finger_tip_index/1 -> Submatrix1/1
  world_frame2vrl_frame1/1(xyz_vrl) -> vrl_xyz_index/1
  Submatrix2/1 -> world_frame2vrl_frame2/1(xyz_0)
  T_finger_tip_middle/1 -> Submatrix2/1
  world_frame2vrl_frame2/1(xyz_vrl) -> vrl_xyz_middle/1
  Submatrix3/1 -> world_frame2vrl_frame3/1(xyz_0)
  T_finger_tip_ring/1 -> Submatrix3/1
  world_frame2vrl_frame3/1(xyz_vrl) -> vrl_xyz_ring/1
  Submatrix4/1 -> world_frame2vrl_frame4/1(xyz_0)
  T_finger_tip_little/1 -> Submatrix4/1
  world_frame2vrl_frame4/1(xyz_vrl) -> vrl_xyz_little/1

== system Simulatore_COMAU_UBHAND_IV_manipolazione_interna/VISUALIZATION/Subsystem4/sphere_xyz1 ==
  xyz_0 : Inport
  Constant : Constant  Value=0
  Constant1 : Constant  Value=0
  Constant2 : Constant  Value=0
  Demux : Demux  Outputs=3
  Display : Display
  Display1 : Display
  Gain : Gain  Gain=-1
  Gain1 : Gain  Gain=-1
  Gain2 : Gain
  Mux : Mux  Inputs=3
  Sum : Sum
  Sum1 : Sum
  Sum2 : Sum
  xyz_vrl : Outport
  wires:
  xyz_0/1 -> Display1/1, Demux/1
  Demux/1 -> Sum/2
  Constant/1 -> Sum/1
  Demux/3 -> Sum2/2
  Constant2/1 -> Sum2/1
  Demux/2 -> Sum1/2
  Constant1/1 -> Sum1/1
  Mux/1 -> xyz_vrl/1, Display/1
  Sum/1 -> Gain/1
  Sum1/1 -> Gain1/1
  Sum2/1 -> Gain2/1
  Gain/1 -> Mux/3
  Gain2/1 -> Mux/2
  Gain1/1 -> Mux/1

== system Simulatore_COMAU_UBHAND_IV_manipolazione_interna/VISUALIZATION/Subsystem4/sphere_xyz3 ==
  xyz_0 : Inport
  Constant : Constant  Value=0
  Constant1 : Constant  Value=0
  Constant2 : Constant  Value=0
  Demux : Demux  Outputs=3
  Display : Display
  Display1 : Display
  Gain : Gain  Gain=-1
  Gain1 : Gain  Gain=-1
  Gain2 : Gain
  Mux : Mux  Inputs=3
  Sum : Sum
  Sum1 : Sum
  Sum2 : Sum
  xyz_vrl : Outport
  wires:
  xyz_0/1 -> Display1/1, Demux/1
  Demux/1 -> Sum/2
  Constant/1 -> Sum/1
  Demux/3 -> Sum2/2
  Constant2/1 -> Sum2/1
  Demux/2 -> Sum1/2
  Constant1/1 -> Sum1/1
  Mux/1 -> xyz_vrl/1, Display/1
  Sum/1 -> Gain/1
  Sum1/1 -> Gain1/1
  Sum2/1 -> Gain2/1
  Gain/1 -> Mux/3
  Gain2/1 -> Mux/2
  Gain1/1 -> Mux/1

== system Simulatore_COMAU_UBHAND_IV_manipolazione_interna/VISUALIZATION/Subsystem4/sphere_xyz4 ==
  xyz_0 : Inport
  Constant : Constant  Value=0
  Constant1 : Constant  Value=0
  Constant2 : Constant  Value=0
  Demux : Demux  Outputs=3
  Display : Display
  Display1 : Display
  Gain : Gain  Gain=-1
  Gain1 : Gain  Gain=-1
  Gain2 : Gain
  Mux : Mux  Inputs=3
  Sum : Sum
  Sum1 : Sum
  Sum2 : Sum
  xyz_vrl : Outport
  wires:
  Gain1/1 -> Mux/1
  Gain2/1 -> Mux/2
  Gain/1 -> Mux/3
  Sum2/1 -> Gain2/1
  Sum1/1 -> Gain1/1
  Sum/1 -> Gain/1
  Mux/1 -> Display/1, xyz_vrl/1
  Constant1/1 -> Sum1/1
  Demux/2 -> Sum1/2
  Constant2/1 -> Sum2/1
  Demux/3 -> Sum2/2
  Constant/1 -> Sum/1
  Demux/1 -> Sum/2
  xyz_0/1 -> Demux/1, Display1/1

== system Simulatore_COMAU_UBHAND_IV_manipolazione_interna/VISUALIZATION/Subsystem5/sphere_xyz1 ==
  xyz_0 : Inport
  Constant : Constant  Value=0
  Constant1 : Constant  Value=0
  Constant2 : Constant  Value=0
  Demux : Demux  Outputs=3
  Display : Display
  Display1 : Display
  Gain : Gain  Gain=-1
  Gain1 : Gain  Gain=-1
  Gain2 : Gain
  Mux : Mux  Inputs=3
  Sum : Sum
  Sum1 : Sum
  Sum2 : Sum
  xyz_vrl : Outport
  wires:
  Gain1/1 -> Mux/1
  Gain2/1 -> Mux/2
  Gain/1 -> Mux/3
  Sum2/1 -> Gain2/1
  Sum1/1 -> Gain1/1
  Sum/1 -> Gain/1
  Mux/1 -> Display/1, xyz_vrl/1
  Constant1/1 -> Sum1/1
  Demux/2 -> Sum1/2
  Constant2/1 -> Sum2/1
  Demux/3 -> Sum2/2
  Constant/1 -> Sum/1
  Demux/1 -> Sum/2
  xyz_0/1 -> Demux/1, Display1/1

== system Simulatore_COMAU_UBHAND_IV_manipolazione_interna/VISUALIZATION/Subsystem6/sphere_xyz1 ==
  xyz_0 : Inport
  Constant : Constant  Value=0
  Constant1 : Constant  Value=0
  Constant2 : Constant  Value=0
  Demux : Demux  Outputs=3
  Display : Display
  Display1 : Display
  Gain : Gain  Gain=-1
  Gain1 : Gain  Gain=-1
  Gain2 : Gain
  Mux : Mux  Inputs=3
  Sum : Sum
  Sum1 : Sum
  Sum2 : Sum
  xyz_vrl : Outport
  wires:
  xyz_0/1 -> Display1/1, Demux/1
  Demux/1 -> Sum/2
  Constant/1 -> Sum/1
  Demux/3 -> Sum2/2
  Constant2/1 -> Sum2/1
  Demux/2 -> Sum1/2
  Constant1/1 -> Sum1/1
  Mux/1 -> xyz_vrl/1, Display/1
  Sum/1 -> Gain/1
  Sum1/1 -> Gain1/1
  Sum2/1 -> Gain2/1
  Gain/1 -> Mux/3
  Gain2/1 -> Mux/2
  Gain1/1 -> Mux/1

== system Simulatore_COMAU_UBHAND_IV_manipolazione_interna/VISUALIZATION/Subsystem9/Index ==
  xyz_0 : Inport
  Constant : Constant  Value=0
  Constant1 : Constant  Value=0
  Constant2 : Constant  Value=0
  Demux : Demux
  Display : Display
  Display1 : Display
  Gain : Gain  Gain=-1
  Gain1 : Gain  Gain=-1
  Gain2 : Gain
  Mux : Mux  Inputs=3
  Sum : Sum
  Sum1 : Sum
  Sum2 : Sum
  xyz_vrl : Outport
  wires:
  Gain1/1 -> Mux/1
  Gain2/1 -> Mux/2
  Gain/1 -> Mux/3
  Sum2/1 -> Gain2/1
  Sum1/1 -> Gain1/1
  Sum/1 -> Gain/1
  Mux/1 -> Display/1, xyz_vrl/1
  Constant1/1 -> Sum1/1
  Demux/2 -> Sum1/2
  Constant2/1 -> Sum2/1
  Demux/3 -> Sum2/2
  Constant/1 -> Sum/1
  Demux/1 -> Sum/2
  xyz_0/1 -> Display1/1, Demux/1

== system Simulatore_COMAU_UBHAND_IV_manipolazione_interna/VISUALIZATION/Subsystem9/Little ==
  xyz_0 : Inport
  Constant : Constant  Value=0
  Constant1 : Constant  Value=0
  Constant2 : Constant  Value=0
  Demux : Demux
  Display : Display
  Display1 : Display
  Gain : Gain  Gain=-1
  Gain1 : Gain  Gain=-1
  Gain2 : Gain
  Mux : Mux  Inputs=3
  Sum : Sum
  Sum1 : Sum
  Sum2 : Sum
  xyz_vrl : Outport
  wires:
  Gain1/1 -> Mux/1
  Gain2/1 -> Mux/2
  Gain/1 -> Mux/3
  Sum2/1 -> Gain2/1
  Sum1/1 -> Gain1/1
  Sum/1 -> Gain/1
  Mux/1 -> Display/1, xyz_vrl/1
  Constant1/1 -> Sum1/1
  Demux/2 -> Sum1/2
  Constant2/1 -> Sum2/1
  Demux/3 -> Sum2/2
  Constant/1 -> Sum/1
  Demux/1 -> Sum/2
  xyz_0/1 -> Display1/1, Demux/1

== system Simulatore_COMAU_UBHAND_IV_manipolazione_interna/VISUALIZATION/Subsystem9/Middle ==
  xyz_0 : Inport
  Constant : Constant  Value=0
  Constant1 : Constant  Value=0
  Constant2 : Constant  Value=0
  Demux : Demux
  Display : Display
  Display1 : Display
  Gain : Gain  Gain=-1
  Gain1 : Gain  Gain=-1
  Gain2 : Gain
  Mux : Mux  Inputs=3
  Sum : Sum
  Sum1 : Sum
  Sum2 : Sum
  xyz_vrl : Outport
  wires:
  Gain1/1 -> Mux/1
  Gain2/1 -> Mux/2
  Gain/1 -> Mux/3
  Sum2/1 -> Gain2/1
  Sum1/1 -> Gain1/1
  Sum/1 -> Gain/1
  Mux/1 -> Display/1, xyz_vrl/1
  Constant1/1 -> Sum1/1
  Demux/2 -> Sum1/2
  Constant2/1 -> Sum2/1
  Demux/3 -> Sum2/2
  Constant/1 -> Sum/1
  Demux/1 -> Sum/2
  xyz_0/1 -> Display1/1, Demux/1

== system Simulatore_COMAU_UBHAND_IV_manipolazione_interna/VISUALIZATION/Subsystem9/Ring ==
  xyz_0 : Inport
  Constant : Constant  Value=0
  Constant1 : Constant  Value=0
  Constant2 : Constant  Value=0
  Demux : Demux
  Display : Display
  Display1 : Display
  Gain : Gain  Gain=-1
  Gain1 : Gain  Gain=-1
  Gain2 : Gain
  Mux : Mux  Inputs=3
  Sum : Sum
  Sum1 : Sum
  Sum2 : Sum
  xyz_vrl : Outport
  wires:
  xyz_0/1 -> Display1/1, Demux/1
  Demux/1 -> Sum/2
  Constant/1 -> Sum/1
  Demux/3 -> Sum2/2
  Constant2/1 -> Sum2/1
  Demux/2 -> Sum1/2
  Constant1/1 -> Sum1/1
  Mux/1 -> xyz_vrl/1, Display/1
  Sum/1 -> Gain/1
  Sum1/1 -> Gain1/1
  Sum2/1 -> Gain2/1
  Gain/1 -> Mux/3
  Gain2/1 -> Mux/2
  Gain1/1 -> Mux/1

== system Simulatore_COMAU_UBHAND_IV_manipolazione_interna/VISUALIZATION/Subsystem9/Thumb ==
  xyz_0 : Inport
  Constant : Constant  Value=0
  Constant1 : Constant  Value=0
  Constant2 : Constant  Value=0
  Demux : Demux
  Display : Display
  Display1 : Display
  Gain : Gain  Gain=-1
  Gain1 : Gain  Gain=-1
  Gain2 : Gain
  Mux : Mux  Inputs=3
  Sum : Sum
  Sum1 : Sum
  Sum2 : Sum
  xyz_vrl : Outport
  wires:
  xyz_0/1 -> Display1/1, Demux/1
  Demux/1 -> Sum/2
  Constant/1 -> Sum/1
  Demux/3 -> Sum2/2
  Constant2/1 -> Sum2/1
  Demux/2 -> Sum1/2
  Constant1/1 -> Sum1/1
  Mux/1 -> xyz_vrl/1, Display/1
  Sum/1 -> Gain/1
  Sum1/1 -> Gain1/1
  Sum2/1 -> Gain2/1
  Gain/1 -> Mux/3
  Gain2/1 -> Mux/2
  Gain1/1 -> Mux/1

== system Simulatore_COMAU_UBHAND_IV_manipolazione_interna/Work Space Control/ /into fingers references ==
  note: THUMB
  note: MIDDLE
  note: RING
  note: LITTLE
  note: 4
  Constant{,1..4} (5 alike) : Constant
  Mux{1..5} (5 alike) : Mux
  x : simulink/Math Operations/Slider Gain  low=0  gain=33.6  high=240
  x2 : simulink/Math Operations/Slider Gain  low=0  gain=111.055  high=116.9
  x3 : simulink/Math Operations/Slider Gain  low=0  gain=116.9  high=116.9
  x4 : simulink/Math Operations/Slider Gain  low=-50  gain=110  high=116.9
  y : simulink/Math Operations/Slider Gain  low=-20  gain=80  high=80
  y2 : simulink/Math Operations/Slider Gain  low=-20  gain=0  high=20
  y3 : simulink/Math Operations/Slider Gain  low=-20  gain=0  high=20
  y4 : simulink/Math Operations/Slider Gain  low=-100  gain=0  high=100
  z : simulink/Math Operations/Slider Gain  low=-30  gain=-30  high=50
  z2 : simulink/Math Operations/Slider Gain  low=0  gain=98  high=200
  z3 : simulink/Math Operations/Slider Gain  low=0  gain=50  high=50
  z4 : simulink/Math Operations/Slider Gain  low=0  gain=48  high=100
  fingers_xyz : Outport
  wires:
  [one] -> x/1
  x/1 -> Mux1/1
  [one] -> y/1
  [one] -> z/1
  [one] -> x2/1
  x2/1 -> Mux2/1
  [one] -> y2/1
  y2/1 -> Mux2/2
  [one] -> z2/1
  z2/1 -> Mux2/3
  [one] -> x3/1
  x3/1 -> Mux3/1
  [one] -> y3/1
  y3/1 -> Mux3/2
  [one] -> z3/1
  z3/1 -> Mux3/3
  [one] -> x4/1
  x4/1 -> Mux4/1
  [one] -> y4/1
  y4/1 -> Mux4/2
  [one] -> z4/1
  z4/1 -> Mux4/3
  Mux4/1 -> Mux5/4
  z/1 -> Mux1/3
  y/1 -> Mux1/2
  Mux5/1 -> fingers_xyz/1
  Constant/1 -> [one]
  Mux1/1 -> Mux5/1
  Mux2/1 -> Mux5/2
  Mux3/1 -> Mux5/3
  Constant1/1 -> Mux1/4
  Constant2/1 -> Mux2/4
  Constant3/1 -> Mux3/4
  Constant4/1 -> Mux4/4

== system Simulatore_COMAU_UBHAND_IV_manipolazione_interna/Work Space Control/Grasp Planner/SCOPES ==
  q_puma : Inport
  q_wrist : Inport  Port=2
  q_fingers : Inport  Port=3
  Demux : Demux  Outputs=5
  Demux1 : Demux  Outputs=5
  Demux2 : Demux  Outputs=6
  Direct_kinematic2 : Subsystem
  Scope : Scope  NumInputPorts=6
      settings: YMin=0~-42.5~5~-30~55~-95  YMax=30~-17.5~40~25~95~-50
  T_0HAND : Display
  T_finger_tip_index : Display
  T_finger_tip_little : Display
  T_finger_tip_middle : Display
  T_finger_tip_ring : Display
  T_finger_tip_thumb : Display
  q[1..2] wrist : Display
  q[1..4] index : Display
  q[1..4] little : Display
  q[1..4] middle : Display
  q[1..4] ring : Display
  q[1..4] thumb : Display
  q[1..6] : Display
  wires:
  Demux/1 -> T_finger_tip_thumb/1
  Demux/2 -> T_finger_tip_index/1
  Demux/3 -> T_finger_tip_middle/1
  Demux/4 -> T_finger_tip_ring/1
  Demux/5 -> T_finger_tip_little/1
  q_puma/1 -> q[1..6]/1, Direct_kinematic2/1(q[1..6]), Demux2/1
  q_wrist/1 -> q[1..2] wrist/1, Direct_kinematic2/2(wrist_angles[1..2])
  Direct_kinematic2/2(fingers_tips_xyz) -> Demux/1
  q_fingers/1 -> Demux1/1, Direct_kinematic2/3(fingers_joints_references)
  Demux1/1 -> q[1..4] thumb/1
  Demux1/2 -> q[1..4] index/1
  Demux1/3 -> q[1..4] middle/1
  Demux1/4 -> q[1..4] ring/1
  Demux1/5 -> q[1..4] little/1
  Direct_kinematic2/1(T0HAND) -> T_0HAND/1
  Demux2/1 -> Scope/1
  Demux2/2 -> Scope/2
  Demux2/3 -> Scope/3
  Demux2/6 -> Scope/6
  Demux2/4 -> Scope/4
  Demux2/5 -> Scope/5

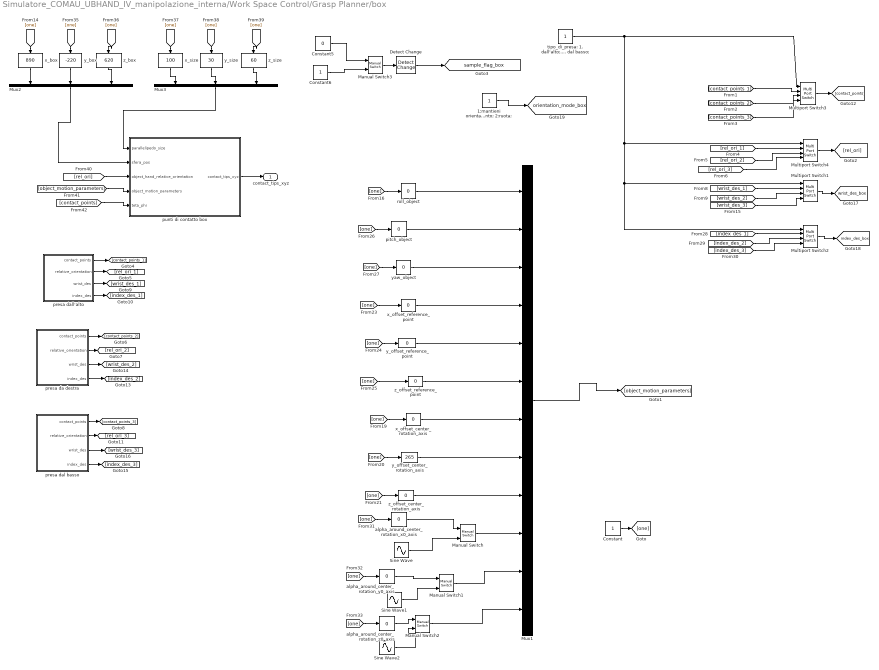
== system Simulatore_COMAU_UBHAND_IV_manipolazione_interna/Work Space Control/Grasp Planner/box ==
  1:mantieni orientamento; 2:ruota; : Constant
  Constant : Constant
  Constant5 : Constant  Value=0
  Constant6 : Constant
  Detect Change : simulink/Logic and Bit Operations/Detect Change  vinit=0  InputProcessing=Inherited  OutDataTypeStr=uint8
  Manual Switch{,1..3} (4 alike) : simulink/Signal Routing/Manual Switch  sw=1  action=0  varsize=off
  Multiport Switch{1..4} (4 alike) : MultiPortSwitch  zeroidx=off
  Mux1 : Mux  Inputs=12
  Mux2 : Mux  Inputs=3
  Mux3 : Mux  Inputs=3
  Sine Wave : Sin  Amplitude=60  Bias=30  SampleTime=0
  Sine Wave1 : Sin  Amplitude=60  Bias=30  SampleTime=0
  Sine Wave2 : Sin  Amplitude=3600  Frequency=0.1  Phase=pi  SampleTime=0
  alpha_around_center_rotation_x0_axis : simulink/Math Operations/Slider Gain  low=-180  gain=0  high=180
  alpha_around_center_rotation_y0_axis : simulink/Math Operations/Slider Gain  low=-180  gain=0  high=180
  alpha_around_center_rotation_z0_axis : simulink/Math Operations/Slider Gain  low=-180  gain=0  high=180
  pitch_object : simulink/Math Operations/Slider Gain  low=-180  gain=0  high=180
  presa da destra : Subsystem
  presa dal basso : Subsystem
  presa dall'alto : Subsystem
  punti di contatto box : Subsystem
  roll_object : simulink/Math Operations/Slider Gain  low=-180  gain=0  high=180
  tipo_di_presa: 1. dall'alto; 2. da destra; 3. dal basso; : Constant
  x_box : simulink/Math Operations/Slider Gain  low=-1000  gain=890  high=2000
  x_offset_center_rotation_axis : simulink/Math Operations/Slider Gain  low=0  gain=0  high=500
  x_offset_reference_point : simulink/Math Operations/Slider Gain  low=0  gain=0  high=500
  x_size : simulink/Math Operations/Slider Gain  low=0  gain=100  high=500
  y_box : simulink/Math Operations/Slider Gain  low=-1000  gain=-220  high=1000
  y_offset_center_rotation_axis : simulink/Math Operations/Slider Gain  low=0  gain=265  high=500
  y_offset_reference_point : simulink/Math Operations/Slider Gain  low=0  gain=0  high=500
  y_size : simulink/Math Operations/Slider Gain  low=0  gain=30  high=500
  yaw_object : simulink/Math Operations/Slider Gain  low=-180  gain=0  high=180
  z_box : simulink/Math Operations/Slider Gain  low=-1000  gain=620  high=2000
  z_offset_center_rotation_axis : simulink/Math Operations/Slider Gain  low=-1000  gain=0  high=500
  z_offset_reference_point : simulink/Math Operations/Slider Gain  low=-1000  gain=0  high=500
  z_size : simulink/Math Operations/Slider Gain  low=0  gain=60  high=500
  contact_tips_xyz : Outport
  tag scope: index_des_box=global, orientation_mode_box=global, sample_flag_box=global, wrist_des_box=global
  wires:
  Mux1/1 -> [object_motion_parameters]
  Constant/1 -> [one]
  tipo_di_presa: 1. dall'alto; 2. da destra; 3. dal basso;/1 -> Multiport Switch3/1, Multiport Switch4/1, Multiport Switch1/1, Multiport Switch2/1
  presa dall'alto/1(contact_points) -> [contact_points_1]
  presa dall'alto/2(relative_orientation) -> [rel_ori_1]
  presa da destra/1(contact_points) -> [contact_points_2]
  presa da destra/2(relative_orientation) -> [rel_ori_2]
  presa dal basso/1(contact_points) -> [contact_points_3]
  presa dal basso/2(relative_orientation) -> [rel_ori_3]
  [rel_ori_3] -> Multiport Switch4/4
  [rel_ori_2] -> Multiport Switch4/3
  [rel_ori_1] -> Multiport Switch4/2
  [contact_points_1] -> Multiport Switch3/2
  [contact_points_2] -> Multiport Switch3/3
  [contact_points_3] -> Multiport Switch3/4
  Multiport Switch3/1 -> [contact_points]
  Multiport Switch4/1 -> [rel_ori]
  presa dall'alto/3(wrist_des) -> [wrist_des_1]
  presa dall'alto/4(index_des) -> [index_des_1]
  presa da destra/3(wrist_des) -> [wrist_des_2]
  presa da destra/4(index_des) -> [index_des_2]
  presa dal basso/3(wrist_des) -> [wrist_des_3]
  presa dal basso/4(index_des) -> [index_des_3]
  [wrist_des_1] -> Multiport Switch1/2
  [wrist_des_2] -> Multiport Switch1/3
  [wrist_des_3] -> Multiport Switch1/4
  [index_des_1] -> Multiport Switch2/2
  [index_des_3] -> Multiport Switch2/4
  [index_des_2] -> Multiport Switch2/3
  Multiport Switch1/1 -> [wrist_des_box]
  Multiport Switch2/1 -> [index_des_box]
  1:mantieni orientamento; 2:ruota;/1 -> [orientation_mode_box]
  [one] -> x_box/1
  x_box/1 -> Mux2/1
  [one] -> y_box/1
  y_box/1 -> Mux2/2
  [one] -> z_box/1
  z_box/1 -> Mux2/3
  Mux2/1 -> punti di contatto box/2(sfera_pos)
  [one] -> x_size/1
  x_size/1 -> Mux3/1
  [one] -> y_size/1
  y_size/1 -> Mux3/2
  [one] -> z_size/1
  Mux3/1 -> punti di contatto box/1(parallelipedo_size)
  [contact_points] -> punti di contatto box/5(teta_phi)
  [object_motion_parameters] -> punti di contatto box/4(object_motion_parameters)
  [rel_ori] -> punti di contatto box/3(object_hand_relative_orientation)
  punti di contatto box/1(contact_tips_xyz) -> contact_tips_xyz/1
  z_size/1 -> Mux3/3
  [one] -> roll_object/1
  [one] -> pitch_object/1
  [one] -> yaw_object/1
  [one] -> alpha_around_center_rotation_x0_axis/1
  [one] -> x_offset_center_rotation_axis/1
  [one] -> y_offset_center_rotation_axis/1
  [one] -> z_offset_center_rotation_axis/1
  [one] -> z_offset_reference_point/1
  [one] -> y_offset_reference_point/1
  [one] -> x_offset_reference_point/1
  roll_object/1 -> Mux1/1
  pitch_object/1 -> Mux1/2
  yaw_object/1 -> Mux1/3
  x_offset_reference_point/1 -> Mux1/4
  y_offset_reference_point/1 -> Mux1/5
  z_offset_reference_point/1 -> Mux1/6
  x_offset_center_rotation_axis/1 -> Mux1/7
  y_offset_center_rotation_axis/1 -> Mux1/8
  z_offset_center_rotation_axis/1 -> Mux1/9
  [one] -> alpha_around_center_rotation_y0_axis/1
  [one] -> alpha_around_center_rotation_z0_axis/1
  alpha_around_center_rotation_x0_axis/1 -> Manual Switch/1
  Sine Wave/1 -> Manual Switch/2
  Manual Switch/1 -> Mux1/10
  alpha_around_center_rotation_y0_axis/1 -> Manual Switch1/1
  Sine Wave1/1 -> Manual Switch1/2
  Manual Switch1/1 -> Mux1/11
  alpha_around_center_rotation_z0_axis/1 -> Manual Switch2/1
  Sine Wave2/1 -> Manual Switch2/2
  Manual Switch2/1 -> Mux1/12
  Constant6/1 -> Manual Switch3/2
  Constant5/1 -> Manual Switch3/1
  Manual Switch3/1 -> Detect Change/1
  Detect Change/1 -> [sample_flag_box]
